simulink model "r_128w_8k_16x_1a_r421"  (Simulink 7.2) (continued)
== system r_128w_8k_16x_1a_r421 == (continued)
      link override convert: arith_type=Unsigned  n_bits=32  bin_pt=0
      mask prompts: io_dir "I/O direction"; arith_type "Data Type"; bitwidth "Data bitwidth"; bin_pt "Data binary point"; sample_period "Sample period"
      mask init:
      | gw_name = clear_name(gcb);
      | config.source = str2func('software_register_init');
      | config.toplevel = gcb;
      | xBlock( config, {io_dir, arith_type, bitwidth, bin_pt, ... 
      |     sample_period, gw_name, latency});
      mask StyleString: popup(From Processor|To Processor),popup(Unsigned|Signed  (2's comp)),edit,edit,edit,edit
  gbe_tx_err_cnt0 : Subsystem  Tag=xps:sw_reg  mask{io_dir=To Processor, arith_type=Unsigned, bitwidth=32, bin_pt=0, sample_period=1, latency=0}
      link override convert: arith_type=Unsigned  n_bits=32  bin_pt=0
      mask prompts: io_dir "I/O direction"; arith_type "Data Type"; bitwidth "Data bitwidth"; bin_pt "Data binary point"; sample_period "Sample period"
      mask init:
      | gw_name = clear_name(gcb);
      | config.source = str2func('software_register_init');
      | config.toplevel = gcb;
      | xBlock( config, {io_dir, arith_type, bitwidth, bin_pt, ... 
      |     sample_period, gw_name, latency});
      mask StyleString: popup(From Processor|To Processor),popup(Unsigned|Signed  (2's comp)),edit,edit,edit,edit
  gpio_tr : Subsystem  mask{cnt_bits=8, time_bits=48}
      mask prompts: cnt_bits "Bits in status counters"; time_bits "No LSBs in time to compare"
  katadc0 : Subsystem  Tag=xps:katadc  AncestorBlock=xps_library/katadc  mask(katadc){adc_brd=adc0, adc_interleave=off, bypass_auto=off, en_gain=on, adc_clk_rate=800, sample_period=1}
      mask prompts: adc_brd "ADC board"; adc_interleave "ADC interleave mode"; bypass_auto "Bypass auto config"; en_gain "Enable gain"; adc_clk_rate "ADC sampling rate (MHz)"; sample_period "Sample period"
      mask init:
      | name = gcb;
      | katadc_init(gcb, ...
      |     'name', name, ...
      |     'adc_brd', adc_brd, ...
      |     'adc_interleave', adc_interleave, ...
      |     'bypass_auto', bypass_auto, ...
      |     'en_gain', en_gain, ...
      |     'adc_clk_rate', adc_clk_rate, ...
      |     'sample_period', sample_period);
      mask StyleString: popup(adc0|adc1),checkbox,checkbox,checkbox,edit,edit
  ld_time_lsw0 : Subsystem  Tag=xps:sw_reg  mask{io_dir=From Processor, arith_type=Unsigned, bitwidth=32, bin_pt=0, sample_period=1, latency=0}
      link override poco_cd_tr0_ld_time_lsb_user_data_out: arith_type=Unsigned  n_bits=32  bin_pt=0  period=1
      link override poco_dram_vacc_r001_cd_tr0_ld_time_lsb_user_data_out: arith_type=Unsigned  n_bits=32  bin_pt=0  period=1
      link override poco_dram_vacc_r002_cd_tr0_ld_time_lsb_user_data_out: arith_type=Unsigned  n_bits=32  bin_pt=0  period=1
      link override poco_dram_vacc_r003_cd_tr0_ld_time_lsb_user_data_out: arith_type=Unsigned  n_bits=32  bin_pt=0  period=1
      link override poco_dram_vacc_r004_cd_tr0_ld_time_lsb_user_data_out: arith_type=Unsigned  n_bits=32  bin_pt=0  period=1
      link override poco_dram_vacc_r005_cd_tr0_ld_time_lsb_user_data_out: arith_type=Unsigned  n_bits=32  bin_pt=0  period=1
      link override poco_dram_vacc_r006_cd_tr0_ld_time_lsb_user_data_out: arith_type=Unsigned  n_bits=32  bin_pt=0  period=1
      link override poco_r006_cd_tr0_ld_time_lsb_user_data_out: arith_type=Unsigned  n_bits=32  bin_pt=0  period=1
      link override poco_red_cd_ld_time_lsb_user_data_out: arith_type=Unsigned  n_bits=32  bin_pt=0  period=1
      link override poco_ref_roach_adc0_cd_ld_time_lsb_user_data_out: arith_type=Unsigned  n_bits=32  bin_pt=0  period=1
      link override poco_ref_roach_reduced_adc0_cd_ld_time_lsb_user_data_out: arith_type=Unsigned  n_bits=32  bin_pt=0  period=1
      link override poco_roach_cd_tr0_ld_time_lsb_user_data_out: arith_type=Unsigned  n_bits=32  bin_pt=0  period=1
      link override poco_roach_r006_cd_tr0_ld_time_lsb_user_data_out: arith_type=Unsigned  n_bits=32  bin_pt=0  period=1
      link override poco_roach_r007_cd_tr0_ld_time_lsb_user_data_out: arith_type=Unsigned  n_bits=32  bin_pt=0  period=1
      link override poco_roach_red_adc0_cd_ld_time_lsb_user_data_out: arith_type=Unsigned  n_bits=32  bin_pt=0  period=1
      link override poco_roach_vacc_r006_cd_tr0_ld_time_lsb_user_data_out: arith_type=Unsigned  n_bits=32  bin_pt=0  period=1
      mask prompts: io_dir "I/O direction"; arith_type "Data Type"; bitwidth "Data bitwidth"; bin_pt "Data binary point"; sample_period "Sample period"
      mask init:
      | gw_name = clear_name(gcb);
      | config.source = str2func('software_register_init');
      | config.toplevel = gcb;
      | xBlock( config, {io_dir, arith_type, bitwidth, bin_pt, ... 
      |     sample_period, gw_name, latency});
      mask StyleString: popup(From Processor|To Processor),popup(Unsigned|Signed  (2's comp)),edit,edit,edit,edit
  ld_time_lsw1 : Subsystem  Tag=xps:sw_reg  mask{io_dir=From Processor, arith_type=Unsigned, bitwidth=32, bin_pt=0, sample_period=1, latency=0}
      link override poco_cd_tr0_ld_time_lsb_user_data_out: arith_type=Unsigned  n_bits=32  bin_pt=0  period=1
      link override poco_dram_vacc_r001_cd_tr0_ld_time_lsb_user_data_out: arith_type=Unsigned  n_bits=32  bin_pt=0  period=1
      link override poco_dram_vacc_r002_cd_tr0_ld_time_lsb_user_data_out: arith_type=Unsigned  n_bits=32  bin_pt=0  period=1
      link override poco_dram_vacc_r003_cd_tr0_ld_time_lsb_user_data_out: arith_type=Unsigned  n_bits=32  bin_pt=0  period=1
      link override poco_dram_vacc_r004_cd_tr0_ld_time_lsb_user_data_out: arith_type=Unsigned  n_bits=32  bin_pt=0  period=1
      link override poco_dram_vacc_r005_cd_tr0_ld_time_lsb_user_data_out: arith_type=Unsigned  n_bits=32  bin_pt=0  period=1
      link override poco_dram_vacc_r006_cd_tr0_ld_time_lsb_user_data_out: arith_type=Unsigned  n_bits=32  bin_pt=0  period=1
      link override poco_r006_cd_tr0_ld_time_lsb_user_data_out: arith_type=Unsigned  n_bits=32  bin_pt=0  period=1
      link override poco_red_cd_ld_time_lsb_user_data_out: arith_type=Unsigned  n_bits=32  bin_pt=0  period=1
      link override poco_ref_roach_adc0_cd_ld_time_lsb_user_data_out: arith_type=Unsigned  n_bits=32  bin_pt=0  period=1
      link override poco_ref_roach_reduced_adc0_cd_ld_time_lsb_user_data_out: arith_type=Unsigned  n_bits=32  bin_pt=0  period=1
      link override poco_roach_cd_tr0_ld_time_lsb_user_data_out: arith_type=Unsigned  n_bits=32  bin_pt=0  period=1
      link override poco_roach_r006_cd_tr0_ld_time_lsb_user_data_out: arith_type=Unsigned  n_bits=32  bin_pt=0  period=1
      link override poco_roach_r007_cd_tr0_ld_time_lsb_user_data_out: arith_type=Unsigned  n_bits=32  bin_pt=0  period=1
      link override poco_roach_red_adc0_cd_ld_time_lsb_user_data_out: arith_type=Unsigned  n_bits=32  bin_pt=0  period=1
      link override poco_roach_vacc_r006_cd_tr0_ld_time_lsb_user_data_out: arith_type=Unsigned  n_bits=32  bin_pt=0  period=1
      mask prompts: io_dir "I/O direction"; arith_type "Data Type"; bitwidth "Data bitwidth"; bin_pt "Data binary point"; sample_period "Sample period"
      mask init:
      | gw_name = clear_name(gcb);
      | config.source = str2func('software_register_init');
      | config.toplevel = gcb;
      | xBlock( config, {io_dir, arith_type, bitwidth, bin_pt, ... 
      |     sample_period, gw_name, latency});
      mask StyleString: popup(From Processor|To Processor),popup(Unsigned|Signed  (2's comp)),edit,edit,edit,edit
  ld_time_msw0 : Subsystem  Tag=xps:sw_reg  mask{io_dir=From Processor, arith_type=Unsigned, bitwidth=32, bin_pt=0, sample_period=1, latency=0}
      link override poco_cd_tr0_ld_time_msb_user_data_out: arith_type=Unsigned  n_bits=32  bin_pt=0  period=1
      link override poco_dram_vacc_r001_cd_tr0_ld_time_msb_user_data_out: arith_type=Unsigned  n_bits=32  bin_pt=0  period=1
      link override poco_dram_vacc_r002_cd_tr0_ld_time_msb_user_data_out: arith_type=Unsigned  n_bits=32  bin_pt=0  period=1
      link override poco_dram_vacc_r003_cd_tr0_ld_time_msb_user_data_out: arith_type=Unsigned  n_bits=32  bin_pt=0  period=1
      link override poco_dram_vacc_r004_cd_tr0_ld_time_msb_user_data_out: arith_type=Unsigned  n_bits=32  bin_pt=0  period=1
      link override poco_dram_vacc_r005_cd_tr0_ld_time_msb_user_data_out: arith_type=Unsigned  n_bits=32  bin_pt=0  period=1
      link override poco_dram_vacc_r006_cd_tr0_ld_time_msb_user_data_out: arith_type=Unsigned  n_bits=32  bin_pt=0  period=1
      link override poco_r006_cd_tr0_ld_time_msb_user_data_out: arith_type=Unsigned  n_bits=32  bin_pt=0  period=1
      link override poco_red_cd_ld_time_msb_user_data_out: arith_type=Unsigned  n_bits=32  bin_pt=0  period=1
      link override poco_ref_roach_adc0_cd_ld_time_msb_user_data_out: arith_type=Unsigned  n_bits=32  bin_pt=0  period=1
      link override poco_ref_roach_reduced_adc0_cd_ld_time_msb_user_data_out: arith_type=Unsigned  n_bits=32  bin_pt=0  period=1
      link override poco_roach_cd_tr0_ld_time_msb_user_data_out: arith_type=Unsigned  n_bits=32  bin_pt=0  period=1
      link override poco_roach_r006_cd_tr0_ld_time_msb_user_data_out: arith_type=Unsigned  n_bits=32  bin_pt=0  period=1
      link override poco_roach_r007_cd_tr0_ld_time_msb_user_data_out: arith_type=Unsigned  n_bits=32  bin_pt=0  period=1
      link override poco_roach_red_adc0_cd_ld_time_msb_user_data_out: arith_type=Unsigned  n_bits=32  bin_pt=0  period=1
      link override poco_roach_vacc_r006_cd_tr0_ld_time_msb_user_data_out: arith_type=Unsigned  n_bits=32  bin_pt=0  period=1
      mask prompts: io_dir "I/O direction"; arith_type "Data Type"; bitwidth "Data bitwidth"; bin_pt "Data binary point"; sample_period "Sample period"
      mask init:
      | gw_name = clear_name(gcb);
      | config.source = str2func('software_register_init');
      | config.toplevel = gcb;
      | xBlock( config, {io_dir, arith_type, bitwidth, bin_pt, ... 
      |     sample_period, gw_name, latency});
      mask StyleString: popup(From Processor|To Processor),popup(Unsigned|Signed  (2's comp)),edit,edit,edit,edit
  ld_time_msw1 : Subsystem  Tag=xps:sw_reg  mask{io_dir=From Processor, arith_type=Unsigned, bitwidth=32, bin_pt=0, sample_period=1, latency=0}
      link override poco_cd_tr0_ld_time_msb_user_data_out: arith_type=Unsigned  n_bits=32  bin_pt=0  period=1
      link override poco_dram_vacc_r001_cd_tr0_ld_time_msb_user_data_out: arith_type=Unsigned  n_bits=32  bin_pt=0  period=1
      link override poco_dram_vacc_r002_cd_tr0_ld_time_msb_user_data_out: arith_type=Unsigned  n_bits=32  bin_pt=0  period=1
      link override poco_dram_vacc_r003_cd_tr0_ld_time_msb_user_data_out: arith_type=Unsigned  n_bits=32  bin_pt=0  period=1
      link override poco_dram_vacc_r004_cd_tr0_ld_time_msb_user_data_out: arith_type=Unsigned  n_bits=32  bin_pt=0  period=1
      link override poco_dram_vacc_r005_cd_tr0_ld_time_msb_user_data_out: arith_type=Unsigned  n_bits=32  bin_pt=0  period=1
      link override poco_dram_vacc_r006_cd_tr0_ld_time_msb_user_data_out: arith_type=Unsigned  n_bits=32  bin_pt=0  period=1
      link override poco_r006_cd_tr0_ld_time_msb_user_data_out: arith_type=Unsigned  n_bits=32  bin_pt=0  period=1
      link override poco_red_cd_ld_time_msb_user_data_out: arith_type=Unsigned  n_bits=32  bin_pt=0  period=1
      link override poco_ref_roach_adc0_cd_ld_time_msb_user_data_out: arith_type=Unsigned  n_bits=32  bin_pt=0  period=1
      link override poco_ref_roach_reduced_adc0_cd_ld_time_msb_user_data_out: arith_type=Unsigned  n_bits=32  bin_pt=0  period=1
      link override poco_roach_cd_tr0_ld_time_msb_user_data_out: arith_type=Unsigned  n_bits=32  bin_pt=0  period=1
      link override poco_roach_r006_cd_tr0_ld_time_msb_user_data_out: arith_type=Unsigned  n_bits=32  bin_pt=0  period=1
      link override poco_roach_r007_cd_tr0_ld_time_msb_user_data_out: arith_type=Unsigned  n_bits=32  bin_pt=0  period=1
      link override poco_roach_red_adc0_cd_ld_time_msb_user_data_out: arith_type=Unsigned  n_bits=32  bin_pt=0  period=1
      link override poco_roach_vacc_r006_cd_tr0_ld_time_msb_user_data_out: arith_type=Unsigned  n_bits=32  bin_pt=0  period=1
      mask prompts: io_dir "I/O direction"; arith_type "Data Type"; bitwidth "Data bitwidth"; bin_pt "Data binary point"; sample_period "Sample period"
      mask init:
      | gw_name = clear_name(gcb);
      | config.source = str2func('software_register_init');
      | config.toplevel = gcb;
      | xBlock( config, {io_dir, arith_type, bitwidth, bin_pt, ... 
      |     sample_period, gw_name, latency});
      mask StyleString: popup(From Processor|To Processor),popup(Unsigned|Signed  (2's comp)),edit,edit,edit,edit
  leds : Subsystem
  man_sync1 : xbsIndex_r4/Slice  nbits=1  boolean_output=on  mode=Lower Bit Location + Width  bit1=0  base1=MSB of Input  bit0=1  base0=LSB of Input  dbl_ovrd=off  block_type=slice
      settings: block_version=10.1.3
  mcount_lsw : Subsystem  Tag=xps:sw_reg  mask{io_dir=To Processor, arith_type=Unsigned, bitwidth=32, bin_pt=0, sample_period=1, latency=0}
      link override convert: arith_type=Unsigned  n_bits=32  bin_pt=0
      mask prompts: io_dir "I/O direction"; arith_type "Data Type"; bitwidth "Data bitwidth"; bin_pt "Data binary point"; sample_period "Sample period"
      mask init:
      | gw_name = clear_name(gcb);
      | config.source = str2func('software_register_init');
      | config.toplevel = gcb;
      | xBlock( config, {io_dir, arith_type, bitwidth, bin_pt, ... 
      |     sample_period, gw_name, latency});
      mask StyleString: popup(From Processor|To Processor),popup(Unsigned|Signed  (2's comp)),edit,edit,edit,edit
  mcount_msw : Subsystem  Tag=xps:sw_reg  mask{io_dir=To Processor, arith_type=Unsigned, bitwidth=32, bin_pt=0, sample_period=1, latency=0}
      link override convert: arith_type=Unsigned  n_bits=32  bin_pt=0
      mask prompts: io_dir "I/O direction"; arith_type "Data Type"; bitwidth "Data bitwidth"; bin_pt "Data binary point"; sample_period "Sample period"
      mask init:
      | gw_name = clear_name(gcb);
      | config.source = str2func('software_register_init');
      | config.toplevel = gcb;
      | xBlock( config, {io_dir, arith_type, bitwidth, bin_pt, ... 
      |     sample_period, gw_name, latency});
      mask StyleString: popup(From Processor|To Processor),popup(Unsigned|Signed  (2's comp)),edit,edit,edit,edit
  packetiser : Subsystem  mask{payload_bits=5, xengine_bits=4, fft_bits=13}
      mask prompts: payload_bits "Payload length in 64bit words (2^?)"; xengine_bits "Number of x-engines (2^?)"; fft_bits "Number of frequencies (2^?)"
      mask help: Package 32 bit data into 64 bits and identify data as coming from antenna 0 or 1
  pfb_fir_real0 : Subsystem  AncestorBlock=casper_library_pfbs/pfb_fir_real  mask(pfb_fir_real){PFBSize=14, TotalTaps=4, WindowType=hamming, n_inputs=2, MakeBiplex=0, BitWidthIn=8, BitWidthOut=18, CoeffBitWidth=18, CoeffDistMem=0, add_latency=1, mult_latency=2, bram_latency=2, conv_latency=2, quantization=Round  (unbiased: +/- Inf), fwidth=1, specify_mult=off, mult_spec=[2                                      2], adder_folding=on, adder_imp=Behavioral}
      mask prompts: PFBSize "Size of PFB: (2^? pnts)"; TotalTaps "Total Number of Taps:"; WindowType "Windowing Function:"; n_inputs "Number of Simultaneous Inputs: (2^?)"; MakeBiplex "Make Biplex"; BitWidthIn "Input Bitwidth:"; BitWidthOut "Output Bitwidth:"; CoeffBitWidth "Coefficient Bitwidth:"; CoeffDistMem "Use Distributed Memory for Coeffs"; add_latency "Add Latency"; mult_latency "Mult Latency"; bram_latency "BRAM Latency"; conv_latency "Convert latency"; quantization "Quantization Behavior"; fwidth "Bin Width Scaling (normal=1)"; specify_mult "Specify multiplier use (behavioral if not specified)"; mult_spec "Multiplier specification (0=core, 1=embedded, 2=behavioural) (left=1st tap)"; adder_folding "Fold adders into DSPs"; adder_imp "Adder implementation"
      mask help:
      | Fold adders into DSPs: Causes adders to be absorbed into DSP blocks (supported in Virtex5)
      | Adder implementation: Cores using Fabric or DSP48 or behavioral HDL
      mask init:
      | pfb_fir_real_init(gcb,...
      |     'PFBSize', PFBSize,...
      |     'TotalTaps', TotalTaps,...
      |     'WindowType', WindowType,...
      |     'n_inputs', n_inputs,...
      |     'MakeBiplex', MakeBiplex,...
      |     'BitWidthIn', BitWidthIn,...
      |     'BitWidthOut', BitWidthOut,...
      |     'CoeffBitWidth', CoeffBitWidth,...
      |     'CoeffDistMem', CoeffDistMem,...
      |     'add_latency', add_latency,...
      |     'mult_latency', mult_latency,...
      |     'bram_latency', bram_latency,...
      |     'conv_latency', conv_latency,...
      |     'quantization', quantization,...
      |     'fwidth', fwidth,...
      |     'specify_mult', specify_mult,...
      |     'mult_spec', mult_spec,...
      |     'adder_folding', adder_folding, ...
      |     'adder_imp', adder_imp);
      settings: AttributesFormatString=taps=4, add_latency=1
      mask Help: eval('xlWeb(''http://casper.berkeley.edu/wiki/Pfb_fir_real'')')
      mask StyleString: edit,edit,popup(bartlett|barthannwin|blackman|blackmanharris|bohamwin|chebwin|flattopwin|gausswin|hamming|hann|kaiser|nuttallwin|parzenwin|rectwin|tukeywin|triang|userwindow),edit,edit,edit,edit,edit,edit,edit,edit,edit,edit,popup(Truncate|Round  (unbiased: +/- Inf)|Round  (unbiased: Even Values)),edit,checkbox,edit,checkbox,popup(Behavioral|Fabric|DSP48)
      mask CallbackString:
      | |%num_taps = eval(get_param(gcb, 'TotalTaps'));
      | %old_vec = eval(get_param(gcb, 'mult_spec'));=-0l
      | 
      | %vec = 2.*ones(1,num_taps);
      | %old_size = length(old_vec);
      | 
      | %if(old_size <= num_taps),
      | %    new_size = old_size;
      | %else,
      | %    new_size = num_taps;
      | %end
      | 
      | %for n = 1:new_size, vec(n) = old_vec(n); end
      | %set_param(gcb, 'mult_spec', mat2str(vec));||||||||||||||%thisVariable = 'specify_mult';
      | %affectedVars = {'mult_spec'};
      | 
      | %thisBlock = gcb();
      | %thisVarState = get_param(thisBlock, thisVariable);
      | %maskNames = get_param(thisBlock, 'MaskNames');
      | %maskEnables = get_param(thisBlock, 'MaskEnables');
      | 
      | %for varName = affectedVars
      | %    varIndex = find(ismember(maskNames, varName));
      | %    maskEnables{varIndex} = thisVarState;
      | %end
      | 
      | %set_param(thisBlock, 'MaskEnables', maskEnables);|||
  pfb_fir_real1 : Subsystem  AncestorBlock=casper_library_pfbs/pfb_fir_real  mask(pfb_fir_real){PFBSize=14, TotalTaps=4, WindowType=hamming, n_inputs=2, MakeBiplex=0, BitWidthIn=8, BitWidthOut=18, CoeffBitWidth=18, CoeffDistMem=0, add_latency=1, mult_latency=2, bram_latency=2, conv_latency=2, quantization=Round  (unbiased: +/- Inf), fwidth=1, specify_mult=off, mult_spec=[2                                      2], adder_folding=on, adder_imp=Behavioral}
      mask prompts: PFBSize "Size of PFB: (2^? pnts)"; TotalTaps "Total Number of Taps:"; WindowType "Windowing Function:"; n_inputs "Number of Simultaneous Inputs: (2^?)"; MakeBiplex "Make Biplex"; BitWidthIn "Input Bitwidth:"; BitWidthOut "Output Bitwidth:"; CoeffBitWidth "Coefficient Bitwidth:"; CoeffDistMem "Use Distributed Memory for Coeffs"; add_latency "Add Latency"; mult_latency "Mult Latency"; bram_latency "BRAM Latency"; conv_latency "Convert latency"; quantization "Quantization Behavior"; fwidth "Bin Width Scaling (normal=1)"; specify_mult "Specify multiplier use (behavioral if not specified)"; mult_spec "Multiplier specification (0=core, 1=embedded, 2=behavioural) (left=1st tap)"; adder_folding "Fold adders into DSPs"; adder_imp "Adder implementation"
      mask help:
      | Fold adders into DSPs: Causes adders to be absorbed into DSP blocks (supported in Virtex5)
      | Adder implementation: Cores using Fabric or DSP48 or behavioral HDL
      mask init: same as r_128w_8k_16x_1a_r421/pfb_fir_real0
      settings: AttributesFormatString=taps=4, add_latency=1
      mask Help: eval('xlWeb(''http://casper.berkeley.edu/wiki/Pfb_fir_real'')')
      mask StyleString: edit,edit,popup(bartlett|barthannwin|blackman|blackmanharris|bohamwin|chebwin|flattopwin|gausswin|hamming|hann|kaiser|nuttallwin|parzenwin|rectwin|tukeywin|triang|userwindow),edit,edit,edit,edit,edit,edit,edit,edit,edit,edit,popup(Truncate|Round  (unbiased: +/- Inf)|Round  (unbiased: Even Values)),edit,checkbox,edit,checkbox,popup(Behavioral|Fabric|DSP48)
      mask CallbackString:
      | |%num_taps = eval(get_param(gcb, 'TotalTaps'));
      | %old_vec = eval(get_param(gcb, 'mult_spec'));=-0l
      | 
      | %vec = 2.*ones(1,num_taps);
      | %old_size = length(old_vec);
      | 
      | %if(old_size <= num_taps),
      | %    new_size = old_size;
      | %else,
      | %    new_size = num_taps;
      | %end
      | 
      | %for n = 1:new_size, vec(n) = old_vec(n); end
      | %set_param(gcb, 'mult_spec', mat2str(vec));||||||||||||||%thisVariable = 'specify_mult';
      | %affectedVars = {'mult_spec'};
      | 
      | %thisBlock = gcb();
      | %thisVarState = get_param(thisBlock, thisVariable);
      | %maskNames = get_param(thisBlock, 'MaskNames');
      | %maskEnables = get_param(thisBlock, 'MaskEnables');
      | 
      | %for varName = affectedVars
      | %    varIndex = find(ismember(maskNames, varName));
      | %    maskEnables{varIndex} = thisVarState;
      | %end
      | 
      | %set_param(thisBlock, 'MaskEnables', maskEnables);|||
  pkt_cont_tvg0 : Subsystem  mask{p_bits=5, fft_bits=13, x_bits=4}
      mask prompts: p_bits "packet size (2^? 64 bit words)"; fft_bits "Number of channels (2^?)"; x_bits "n_x_engines (2^?)"
  pol_pack0 : Subsystem
  pol_pack1 : Subsystem
  posedge{1..2,23..24} (4 alike) : casper_library_misc/posedge  Tag=Rising Edge Detector  SystemSampleTime=-1
  pps_count : Subsystem  Tag=xps:sw_reg  mask{io_dir=To Processor, arith_type=Unsigned, bitwidth=32, bin_pt=0, sample_period=1, latency=0}
      link override convert: arith_type=Unsigned  n_bits=32  bin_pt=0
      mask prompts: io_dir "I/O direction"; arith_type "Data Type"; bitwidth "Data bitwidth"; bin_pt "Data binary point"; sample_period "Sample period"
      mask init:
      | gw_name = clear_name(gcb);
      | config.source = str2func('software_register_init');
      | config.toplevel = gcb;
      | xBlock( config, {io_dir, arith_type, bitwidth, bin_pt, ... 
      |     sample_period, gw_name, latency});
      mask StyleString: popup(From Processor|To Processor),popup(Unsigned|Signed  (2's comp)),edit,edit,edit,edit
  quant0 : Subsystem  mask{channel_bits=13, din_bit_width=18, din_bin_pt=17, coeff_bit_width=16, coeff_bin_pt=1, dout_bit_width=4, dout_bin_pt=3, init_val=4, overflow=Saturate, quantization=Round  (unbiased: even values), conv_latency=2, add_latency=1, mult_latency=2, bram_latency=2}
      mask prompts: channel_bits "Number channels (2^?)"; din_bit_width "Data input bit width"; din_bin_pt "Data input binary point"; coeff_bit_width "Coefficient bit width"; coeff_bin_pt "Coefficient binary point"; dout_bit_width "Data out bit width"; dout_bin_pt "Data out binary point"; init_val "Initial value"; overflow "Overflow behaviour"; quantization "Quantization Behaviour"; conv_latency "Conversion latency"; add_latency "Adder latency"; mult_latency "Multiplier latency"; bram_latency "BRAM latency"
      mask init:
      | blk = gcb;
      | set_param([blk,'/convert_of0'], ...
      |     'bit_width_i', num2str(din_bit_width+coeff_bit_width+1), ...
      |     'binary_point_i', num2str(din_bin_pt+coeff_bin_pt), ...
      |     'bit_width_o', num2str(dout_bit_width), 'binary_point_o', num2str(dout_bin_pt), ...
      |     'latency', num2str(conv_latency), 'overflow', overflow, ...
      |     'quantization', quantization);
      | set_param([blk,'/convert_of1'], ...
      |     'bit_width_i', num2str(din_bit_width+coeff_bit_width+1), ...
      |     'binary_point_i', num2str(din_bin_pt+coeff_bin_pt), ...
      |     'bit_width_o', num2str(dout_bit_width), 'binary_point_o', num2str(dout_bin_pt), ...
      |     'latency', num2str(conv_latency), 'overflow', overflow, ...
      |     'quantization', quantization);
      | set_param([blk,'/convert_of2'], ...
      |     'bit_width_i', num2str(din_bit_width+coeff_bit_width+1), ...
      |     'binary_point_i', num2str(din_bin_pt+coeff_bin_pt), ...
      |     'bit_width_o', num2str(dout_bit_width), 'binary_point_o', num2str(dout_bin_pt), ...
      |     'latency', num2str(conv_latency), 'overflow', overflow, ...
      |     'quantization', quantization);
      | set_param([blk,'/convert_of3'], ...
      |     'bit_width_i', num2str(din_bit_width+coeff_bit_width+1), ...
      |     'binary_point_i', num2str(din_bin_pt+coeff_bin_pt), ...
      |     'bit_width_o', num2str(dout_bit_width), 'binary_point_o', num2str(dout_bin_pt), ...
      |     'latency', num2str(conv_latency), 'overflow', overflow, ...
      |     'quantization', quantization);
      mask StyleString: edit,edit,edit,edit,edit,edit,edit,edit,popup(Wrap|Saturate|Error),popup(Truncate|Round  (unbiased: +/- Inf)|Round  (unbiased: even values)),edit,edit,edit,edit
  quant1 : Subsystem  mask{channel_bits=13, din_bit_width=18, din_bin_pt=17, coeff_bit_width=16, coeff_bin_pt=1, dout_bit_width=4, dout_bin_pt=3, init_val=4, overflow=Saturate, quantization=Round  (unbiased: even values), conv_latency=2, add_latency=1, mult_latency=2, bram_latency=2}
      mask prompts: channel_bits "Number channels (2^?)"; din_bit_width "Data input bit width"; din_bin_pt "Data input binary point"; coeff_bit_width "Coefficient bit width"; coeff_bin_pt "Coefficient binary point"; dout_bit_width "Data out bit width"; dout_bin_pt "Data out binary point"; init_val "Initial value"; overflow "Overflow behaviour"; quantization "Quantization Behaviour"; conv_latency "Conversion latency"; add_latency "Adder latency"; mult_latency "Multiplier latency"; bram_latency "BRAM latency"
      mask init: same as r_128w_8k_16x_1a_r421/quant0
      mask StyleString: edit,edit,edit,edit,edit,edit,edit,edit,popup(Wrap|Saturate|Error),popup(Truncate|Round  (unbiased: +/- Inf)|Round  (unbiased: even values)),edit,edit,edit,edit
  rcs : Subsystem  AncestorBlock=casper_library_misc/rcs  mask(rcs){app_src=git, lib_src=git, user_val=421}
      mask prompts: app_src "Application revision info to capture"; lib_src "CASPER libraries revision info to capture"; user_val "User specified value"
      mask help:
      | Embeds revision control information for the user design and libraries.
      | On failure to locate specified revision information, falls back to
      | timestamps (current time for libraries).
      | A user specified value is also stored.
      mask init:
      | rcs_init(gcb, ...
      |     'app_src', app_src, ...
      |     'lib_src', lib_src);
      mask StyleString: popup(timestamp|git|svn),popup(timestamp|git|svn),edit
  scopes : Subsystem
  snap_gbe_tx0 : Subsystem  AncestorBlock=casper_library_scopes/snapshot  mask(snapshot){storage=bram, dram_dimm=1, dram_clock=200, nsamples=8, data_width=128, offset=on, circap=off, value=off, use_dsp48=off}
      mask prompts: storage "Storage medium"; dram_dimm "DRAM dimm"; dram_clock "DRAM clock rate"; nsamples "Number of Samples (2^?)"; data_width "Data width"; offset "Start delay support"; circap "Circular capture support"; value "Extra value capture support"; use_dsp48 "Use DSP48s to implement counters"
      mask help:
      | Standard snap block, but with the following optional extras;
      |  1) Optionally delay the fabric trigger by a user-specified number of valids.
      |  2) Capture continuously after a trigger until a stop pulse is received. Data is captured into BRAM continuously, with old data being overwritten until a stop command is received. Then stop capturing when memory is filled. This enables you to get some data before and some data after the stop pulse. Use the 'tr_en_cnt' register to determine where you are in your vector. 'Addr' register's MSb now indicates 'busy capturing'.
      |  3) Optionally capture a value on an input port to a register at the same time as the first data sample.
      |  4) Implement counters using DSP48Es to save resources.
      mask init: same as r_128w_8k_16x_1a_r421/adc_snap0
      mask StyleString: popup(bram|dram),popup(1|2|3|4),popup(200|250|266|300|333),edit,popup(8|16|32|64|128),checkbox,checkbox,checkbox,checkbox
      mask CallbackString: snapshot_callback();||||||||
  timing : Subsystem  mask{reset_period=1, sync_gen_bits=28}
      mask prompts: reset_period "Master reset time (sync pulses)"; sync_gen_bits "Size of sync generator counter (bits)"
  trig0 : Subsystem  mask{mult_latency=3}
      mask prompts: mult_latency "Multiplier latency"
  trig1 : Subsystem  mask{mult_latency=3}
      mask prompts: mult_latency "Multiplier latency"
  trig_level : Subsystem  Tag=xps:sw_reg  mask{io_dir=From Processor, arith_type=Unsigned, bitwidth=32, bin_pt=0, sample_period=1, latency=0}
      link override convert: arith_type=Unsigned  n_bits=32  bin_pt=0
      link override r_spec_2048_r102_cnt_rst_user_data_out: arith_type=Unsigned  n_bits=32  bin_pt=0  period=1
      link override r_spec_2048_r102_nosim_cnt_rst_user_data_out: arith_type=Unsigned  n_bits=32  bin_pt=0  period=1
      link override r_spec_2048_r103_cnt_rst_user_data_out: arith_type=Unsigned  n_bits=32  bin_pt=0  period=1
      link override r_spec_2048_r104_cnt_rst_user_data_out: arith_type=Unsigned  n_bits=32  bin_pt=0  period=1
      link override r_spec_2048_r104_test0_cnt_rst_user_data_out: arith_type=Unsigned  n_bits=32  bin_pt=0  period=1
      link override r_spec_2048_r104_test_cnt_rst_user_data_out: arith_type=Unsigned  n_bits=32  bin_pt=0  period=1
      link override r_spec_test0_cnt_rst_user_data_out: arith_type=Unsigned  n_bits=32  bin_pt=0  period=1
      link override r_spec_test4_cnt_rst_user_data_out: arith_type=Unsigned  n_bits=32  bin_pt=0  period=1
      link override roach_spec_no10gbe_sync_cnt_rst_user_data_out: arith_type=Unsigned  n_bits=32  bin_pt=0  period=1
      link override roach_spec_rst_cnt_user_data_out: arith_type=Unsigned  n_bits=32  bin_pt=0  period=1
      link override roach_spec_rst_sync_cnt_user_data_out: arith_type=Unsigned  n_bits=32  bin_pt=0  period=1
      mask prompts: io_dir "I/O direction"; arith_type "Data Type"; bitwidth "Data bitwidth"; bin_pt "Data binary point"; sample_period "Sample period"
      mask init:
      | gw_name = clear_name(gcb);
      | config.source = str2func('software_register_init');
      | config.toplevel = gcb;
      | xBlock( config, {io_dir, arith_type, bitwidth, bin_pt, ... 
      |     sample_period, gw_name, latency});
      mask StyleString: popup(From Processor|To Processor),popup(Unsigned|Signed  (2's comp)),edit,edit,edit,edit
  tvg_tr : Subsystem  mask{cnt_bits=8, time_bits=48}
      mask prompts: cnt_bits "Bits in status counters"; time_bits "No LSBs in time to compare"
  xaui_pack0 : Subsystem  mask{payload_len=32, xeng_bits=4, chan_bits=13, skip_xeng_bits=1}
      mask prompts: payload_len "Payload Length (in 64 bit words)"; xeng_bits "Number of X engines (2^?)"; chan_bits "Number of Frequency channels (2^?)"; skip_xeng_bits "X engines per IP address (2^?)"
      mask help: Packetizes data coming in over a XAUI interface. A packet consists of a 64 bit header (48 bits of "mcnt" and 16 bits of antenna), followed by 64 x "payload" bits of payload. "Mcnt" (master count) is a counter which keeps track of how many packets have been transmitted since the last "mrst".
      mask init:
      | packet_len = payload_len + 1;
      | packet_bits = ceil(log2(packet_len));
      | payload_bits = ceil(log2(payload_len));
  wires:
  [adc_sync0] -> Logical3/2
  timing/2(sync) -> [sync]
  Logical3/1 -> timing/4(pps_in), [pps]
  Concat/1 -> timing/2(data_in)
  Constant9/1 -> fft_shift0/1(sim_reg_in)
  fft_shift0/1(reg_in) -> Slice6/1
  a0p0_1st_shift/2(dout1) -> fft_wideband_real0/3(in0)
  a0p0_1st_shift/3(dout2) -> fft_wideband_real0/4(in1)
  a0p0_1st_shift/4(dout3) -> fft_wideband_real0/5(in2)
  a0p0_1st_shift/5(dout4) -> fft_wideband_real0/6(in3)
  Slice6/1 -> fft_wideband_real0/2(shift)
  a0p0_1st_shift/1(sync_out) -> fft_wideband_real0/1(sync)
  fft_wideband_real0/1(sync_out) -> fd_local_time/2(sync), Delay20/1
  fft_wideband_real0/4(of) -> [fft_or0]
  pfb_fir_real1/2(pol1_out1) -> a0p1_1st_shift/2(din1)
  pfb_fir_real1/3(pol1_out2) -> a0p1_1st_shift/3(din2)
  pfb_fir_real1/4(pol1_out3) -> a0p1_1st_shift/4(din3)
  pfb_fir_real1/5(pol1_out4) -> a0p1_1st_shift/5(din4)
  a0p1_1st_shift/2(dout1) -> fft_wideband_real1/3(in0)
  a0p1_1st_shift/3(dout2) -> fft_wideband_real1/4(in1)
  a0p1_1st_shift/4(dout3) -> fft_wideband_real1/5(in2)
  a0p1_1st_shift/5(dout4) -> fft_wideband_real1/6(in3)
  pfb_fir_real1/1(sync_out) -> a0p1_1st_shift/1(sync_in)
  a0p1_1st_shift/1(sync_out) -> fft_wideband_real1/1(sync)
  [sync] -> pfb_fir_real1/1(sync)
  bus_expand5/1(msb_out4) -> pfb_fir_real1/2(pol1_in1)
  bus_expand5/2(out3) -> pfb_fir_real1/3(pol1_in2)
  bus_expand5/3(out2) -> pfb_fir_real1/4(pol1_in3)
  bus_expand5/4(lsb_out1) -> pfb_fir_real1/5(pol1_in4)
  bus_expand7/1(msb_out4) -> pfb_fir_real0/2(pol1_in1)
  bus_expand7/2(out3) -> pfb_fir_real0/3(pol1_in2)
  bus_expand7/3(out2) -> pfb_fir_real0/4(pol1_in3)
  bus_expand7/4(lsb_out1) -> pfb_fir_real0/5(pol1_in4)
  quant0/2(dout0) -> Concat7/1
  quant0/3(dout1) -> Concat7/2
  quant0/1(sync_out) -> [quant0_sync]
  quant0/4(of) -> [quant_or0]
  Concat7/1 -> [quant0_data]
  timing/1(armed) -> Logical14/2, [armed]
  Logical14/1 -> [mrst]
  [mrst] -> cd_local_time/1(reset)
  [sync] -> cd_local_time/2(sync)
  cd_local_time/1(time) -> [cd_local_time]
  [sys_rst] -> Logical14/1
  [man_sync] -> Logical3/1
  [arm] -> timing/1(arm)
  Constant2/1 -> timing/3(sync_period)
  pfb_fir_real0/2(pol1_out1) -> a0p0_1st_shift/2(din1)
  pfb_fir_real0/3(pol1_out2) -> a0p0_1st_shift/3(din2)
  pfb_fir_real0/4(pol1_out3) -> a0p0_1st_shift/4(din3)
  pfb_fir_real0/5(pol1_out4) -> a0p0_1st_shift/5(din4)
  pfb_fir_real0/1(sync_out) -> a0p0_1st_shift/1(sync_in)
  Constant33/1 -> ld_time_msw0/1(sim_reg_in)
  Constant29/1 -> ld_time_lsw0/1(sim_reg_in)
  ld_time_msw0/1(reg_in) -> Slice73/1, Slice7/1
  Slice73/1 -> Concat9/1
  ld_time_lsw0/1(reg_in) -> Concat9/2
  fstatus0/1(sim_reg_out) -> Terminator9/1
  Constant18/1 -> control/1(sim_reg_in)
  control/1(reg_in) -> Delay6/1
  Delay6/1 -> Slice78/1, man_sync1/1, arm1/1, arm2/1, arm8/1, arm10/1, arm6/1, arm7/1, arm5/1, arm4/1, arm3/1, arm11/1, arm9/1
  Slice78/1 -> [sys_rst]
  man_sync1/1 -> posedge1/1
  posedge1/1 -> [man_sync]
  arm1/1 -> posedge24/1
  posedge24/1 -> [arm]
  arm2/1 -> posedge23/1
  posedge23/1 -> [clr_status]
  Counter1/1 -> Register19/1
  Register19/1 -> clk_frequency/1(reg_out), Relational/1, Relational1/1
  clk_frequency/1(sim_reg_out) -> Terminator10/1
  pps_count/1(sim_reg_out) -> Terminator11/1
  [mrst] -> Counter3/1
  [cd_local_time] -> Delay7/1
  mcount_msw/1(sim_reg_out) -> Terminator12/1
  mcount_lsw/1(sim_reg_out) -> Terminator13/1
  Slice/1 -> mcount_lsw/1(reg_out)
  Slice1/1 -> mcount_msw/1(reg_out)
  fstatus1/1(sim_reg_out) -> Terminator2/1
  Constant32/1 -> coarse_delay0/1(sim_reg_in)
  Constant8/1 -> a0_fd0/1(sim_reg_in)
  Constant4/1 -> a1_fd0/1(sim_reg_in)
  a0_fd0/1(reg_in) -> Slice3/1, Slice2/1
  Slice4/1 -> Concat22/4
  Slice5/1 -> Concat22/5
  Constant27/1 -> adc_ctrl0/1(sim_reg_in)
  adc_ctrl0/1(reg_in) -> Slice29/1, Slice30/1
  Logical/1 -> edge_detect1/1
  edge_detect1/1 -> [adc_sync0]
  [mrst] -> fd_local_time/1(reset)
  fd_local_time/1(time) -> tvg_tr/4(current_time), Delay24/1
  [armed] -> Concat21/1
  Counter3/1 -> Concat21/2
  Concat21/1 -> pps_count/1(reg_out)
  Constant40/1 -> fft_shift1/1(sim_reg_in)
  fft_shift1/1(reg_in) -> Slice26/1
  Slice26/1 -> fft_wideband_real1/2(shift)
  calc_adc_sum_sq/1(sum_sq) -> Delay8/1
  decat0/1(Out1) -> calc_adc_sum_sq/1(In1)
  decat0/2(Out2) -> calc_adc_sum_sq/2(In2)
  decat0/3(Out3) -> calc_adc_sum_sq/3(In3)
  decat0/4(Out4) -> calc_adc_sum_sq/4(In4)
  [adc0] -> decat0/1(In1)
  adc_sum_sq0/1(sim_reg_out) -> Terminator/1
  [adc1] -> decat1/1(In1)
  Constant48/1 -> adc_ctrl1/1(sim_reg_in)
  adc_ctrl1/1(reg_in) -> Slice34/1, Slice33/1
  calc_adc_sum_sq1/1(sum_sq) -> Delay12/1
  decat1/1(Out1) -> calc_adc_sum_sq1/1(In1)
  decat1/2(Out2) -> calc_adc_sum_sq1/2(In2)
  decat1/3(Out3) -> calc_adc_sum_sq1/3(In3)
  decat1/4(Out4) -> calc_adc_sum_sq1/4(In4)
  adc_sum_sq1/1(sim_reg_out) -> Terminator25/1
  Constant3/1 -> board_id/1(sim_reg_in)
  board_id/1(reg_in) -> [board_id]
  arm3/1 -> posedge2/1
  posedge2/1 -> [tvg_en]
  arm4/1 -> [ct_tvg]
  arm5/1 -> [packetiser_tvg]
  arm6/1 -> [fd_fs_tvg]
  [clr_status] -> Subsystem6/1(clr)
  [xaui_ldn0] -> Subsystem6/2(xuai_ldn)
  [xaui_of0] -> Subsystem6/3(xaui_of)
  [ct_err0] -> Subsystem6/4(ct_err)
  [adc_or0] -> Subsystem6/5(adc_or)
  [fft_or0] -> Subsystem6/6(fft_or)
  [quant_or0] -> Subsystem6/7(quant_or)
  Counter8/1 -> Slice13/1
  Constant41/1 -> eq0/2(data_in)
  Constant46/1 -> eq0/3(we)
  arm7/1 -> [adc_tvg]
  pol_pack0/1(Out1) -> [adc0]
  pol_pack1/1(Out1) -> [adc1]
  coarse_delay0/1(reg_in) -> Slice38/1
  Slice2/1 -> Concat22/1
  Slice3/1 -> Concat22/2
  [mrst] -> packetiser/4(mrst_in)
  ct/4(ct_err) -> [ct_err0]
  X-engine reorder/1(sync_out) -> Recombine channels/1(sync_in)
  X-engine reorder/2(out0) -> Recombine channels/2(channel0)
  X-engine reorder/3(out1) -> Recombine channels/3(channel1)
  Recombine channels/1(sync_out) -> ct/1(sync)
  Recombine channels/2(p0) -> ct/2(p0)
  Recombine channels/3(p1) -> ct/3(p1)
  ct_tvg/1(sync_out) -> Split channels/1(sync_in)
  ct_tvg/2(p0) -> Split channels/2(p0)
  Split channels/1(sync_out) -> X-engine reorder/1(sync)
  ct_tvg/3(p1) -> Split channels/3(p1)
  Split channels/2(even) -> X-engine reorder/2(data0)
  Split channels/3(odd) -> X-engine reorder/3(data1)
  [board_id] -> packetiser/5(ant_base)
  [quant0_sync] -> ct_tvg/2(sync_in)
  [quant0_data] -> ct_tvg/3(p0_in)
  [quant1_data] -> ct_tvg/4(p1_in)
  [ct_tvg] -> Register/1
  [tvg_en] -> Register/2
  Register/1 -> ct_tvg/1(enable)
  fft_wideband_real0/2(out0) -> Delay22/1
  fft_wideband_real0/3(out1) -> Delay21/1
  fft_wideband_real1/1(sync_out) -> Delay25/1
  fft_wideband_real1/2(out0) -> Delay26/1
  fft_wideband_real1/3(out1) -> Delay27/1
  ct/1(sync_out) -> pkt_cont_tvg0/2(sync)
  ct/2(data_valid) -> pkt_cont_tvg0/3(dv)
  pkt_cont_tvg0/1(sync_out) -> packetiser/1(sync_in)
  pkt_cont_tvg0/2(dv_out) -> packetiser/2(data_valid)
  pkt_cont_tvg0/3(dout) -> packetiser/3(data_in)
  [tvg_en] -> Register1/2
  [packetiser_tvg] -> Register1/1
  Register1/1 -> pkt_cont_tvg0/1(use_tvg)
  Register2/1 -> fd_fs_tvg0/3(use_tvg), fd_fs_tvg1/1(use_tvg)
  katadc0/5(or0) -> [adc_or0]
  katadc0/1(data0_0) -> pol_pack0/1(In1)
  katadc0/2(data0_1) -> pol_pack0/2(In2)
  katadc0/3(data0_2) -> pol_pack0/3(In3)
  katadc0/4(data0_3) -> pol_pack0/4(In4)
  katadc0/6(data1_0) -> pol_pack1/1(In1)
  katadc0/7(data1_1) -> pol_pack1/2(In2)
  katadc0/8(data1_2) -> pol_pack1/3(In3)
  katadc0/9(data1_3) -> pol_pack1/4(In4)
  katadc0/10(or1) -> [adc_or1]
  katadc0/15(data_valid) -> Terminator1/1
  Pulse Generator2/1 -> katadc0/1(sim_data0)
  Pulse Generator/1 -> katadc0/2(sim_data1)
  Pulse Generator1/1 -> katadc0/3(sim_sync)
  Constant/1 -> katadc0/4(sim_data_valid)
  xaui_pack0/6(sync) -> Delay31/1(d)
  xaui_pack0/7(mrst) -> Delay34/1(d)
  xaui_pack0/5(discard) -> Delay33/1(d)
  [gbe_base_ip] -> xaui_pack0/5(base_ip)
  xaui_pack0/1(dout) -> Delay9/1(d)
  xaui_pack0/2(valid_out) -> Delay23/1(d)
  xaui_pack0/3(dest_ip) -> Delay10/1(d)
  xaui_pack0/4(eof) -> Delay11/1(d)
  Delay1/1(q) -> xaui_pack0/1(din)
  Delay3/1(q) -> xaui_pack0/2(oob)
  Delay4/1(q) -> xaui_pack0/3(valid_in)
  Delay5/1(q) -> xaui_pack0/4(linkdown_in)
  Delay31/1(q) -> [xaui_sync]
  Delay33/1(q) -> [xaui_pkt_err]
  Delay34/1(q) -> [xaui_mrst]
  [gbe_enable] -> Logical1/2
  xaui_pack0/8(hdr) -> Terminator8/1
  xaui_pack0/9(hdr_valid) -> Terminator7/1
  [xaui_data] -> Delay1/1(d)
  [xaui_oob] -> Delay3/1(d)
  [xaui_valid] -> Delay4/1(d)
  [xaui_link_dn] -> Delay5/1(d)
  Delay9/1(q) -> [xaui_pkt_data]
  Delay23/1(q) -> [xaui_pkt_valid]
  Delay10/1(q) -> [xaui_pkt_ip]
  Delay11/1(q) -> [xaui_pkt_eof]
  [xaui_pkt_valid] -> Logical1/1
  Logical2/1 -> [gbe_rst_int]
  [gbe_rst] -> Logical2/2
  Constant20/1 -> Logical2/1
  Logical1/1 -> [gbe_txvld]
  [xaui_pkt_data] -> [gbe_txd]
  [xaui_pkt_ip] -> [gbe_txip]
  [xaui_pkt_eof] -> [gbe_txeof]
  [xaui_pkt_err] -> [gbe_tx_discard]
  [gbe_rst_int] -> gbe0/1
  [gbe_txd] -> gbe0/2
  [gbe_txvld] -> gbe0/3
  [gbe_txip] -> gbe0/4
  [gbe_txport] -> gbe0/5
  [gbe_txeof] -> gbe0/6
  [gbe_rxack] -> gbe0/7
  Constant5/1 -> [gbe_rxack]
  [gbe_rxack] -> gbe0/8
  gbe0/1 -> [gbe_up], Inverter/1
  gbe0/2 -> [gbe_rx]
  gbe0/3 -> [gbe_tx]
  gbe0/4 -> [gbe_tx_full]
  gbe0/5 -> [gbe_tx_over], [xaui_of0]
  gbe0/12 -> [gbe_rx_over]
  gbe0/11 -> [gbe_rx_bad]
  gbe0/6 -> [gbe_rxdata]
  gbe0/7 -> [gbe_rxvld]
  gbe0/8 -> [gbe_rxip]
  gbe0/9 -> [gbe_rxport]
  gbe0/10 -> [gbe_rxeof]
  err_clear_sim4/1 -> gbe_port/1(sim_reg_in)
  gbe_port/1(reg_in) -> Delay71/1(d)
  err_clear_sim3/1 -> gbe_ip0/1(sim_reg_in)
  gbe_ip0/1(reg_in) -> Delay72/1(d)
  Delay71/1(q) -> [gbe_txport]
  Delay72/1(q) -> [gbe_base_ip]
  packetiser/1(data_out) -> [xaui_data]
  packetiser/2(oob) -> [xaui_oob]
  packetiser/3(valid_out) -> [xaui_valid]
  Constant7/1 -> [xaui_link_dn]
  arm8/1 -> Delay100/1(d)
  arm9/1 -> Delay2/1(d)
  Delay100/1(q) -> [gbe_enable]
  Delay2/1(q) -> [gbe_rst]
  Inverter/1 -> [xaui_ldn0]
  gbe_err/1(tx_cnt) -> Delay14/1(d)
  [clr_status] -> gbe_err/1(rst)
  gbe_err/2(err_cnt) -> Delay64/1(d)
  gbe_tx_err_cnt0/1(sim_reg_out) -> Terminator4/1
  Delay64/1(q) -> gbe_tx_err_cnt0/1(reg_out)
  [gbe_tx_discard] -> gbe_err/2(discard)
  [gbe_txeof] -> gbe_err/3(eof)
  [gbe_txvld] -> gbe_err/4(valid)
  Subsystem1/1(status) -> fstatus1/1(reg_out)
  arm10/1 -> [fancy_en]
  fft_wideband_real1/4(of) -> [fft_or1]
  [adc0] -> decat2/1(i1)
  decat2/1(o1) -> auto_shutoff0/1(In1)
  decat2/2(o2) -> auto_shutoff0/2(In2)
  decat2/3(o3) -> auto_shutoff0/3(In3)
  decat2/4(o4) -> auto_shutoff0/4(In4)
  [clr_status] -> auto_shutoff0/5(rst)
  auto_shutoff0/1(en) -> [adc_bad0]
  katadc0/11(sync0) -> Concat8/1
  katadc0/12(sync1) -> Concat8/2
  katadc0/13(sync2) -> Concat8/3
  katadc0/14(sync3) -> Concat8/4
  Concat8/1 -> [sync_raw0]
  Slice35/1 -> Logical/1
  Slice36/1 -> Logical/2
  Slice40/1 -> Logical/3
  Slice45/1 -> Logical/4
  [sync_raw0] -> Slice35/1, Slice45/1, Slice40/1, Slice36/1
  [sync_val0] -> Subsystem6/8(sync_val)
  [adc1] -> decat3/1(i1)
  decat3/1(o1) -> auto_shutoff1/1(In1)
  decat3/2(o2) -> auto_shutoff1/2(In2)
  decat3/3(o3) -> auto_shutoff1/3(In3)
  decat3/4(o4) -> auto_shutoff1/4(In4)
  [clr_status] -> auto_shutoff1/5(rst)
  auto_shutoff1/1(en) -> [adc_bad1]
  arm11/1 -> [adc_protect_disable]
  [adc_bad_latch0] -> Inverter1/1
  Inverter1/1 -> Logical6/1
  [adc_protect_disable] -> Logical6/2
  Logical8/1 -> [adc_en0], katadc0/5(en0)
  Logical6/1 -> Logical8/1
  Slice29/1 -> Logical8/2
  Slice30/1 -> katadc0/6(atten0)
  [adc_bad_latch1] -> Inverter2/1
  Inverter2/1 -> Logical9/1
  [adc_protect_disable] -> Logical9/2
  Logical9/1 -> Logical10/1
  Slice33/1 -> Logical10/2
  Logical10/1 -> [adc_en1], katadc0/7(en1)
  Slice34/1 -> katadc0/8(atten1)
  [mrst] -> edge_detect/1(in)
  edge_detect/1(out) -> Delay/1
  [mrst] -> fd_tr0/1(rst)
  Logical12/1 -> fd_tr0/3(arm)
  [fd_local_time] -> fd_tr0/4(current_time)
  Slice38/1 -> AddSub/1
  [sync_val0] -> AddSub/2
  AddSub/1 -> Concat22/3
  Constant25/1 -> trig_level/1(sim_reg_in)
  trig_level/1(reg_in) -> trig0/3(trig_pnt), trig1/3(trig_pnt)
  [data_adc1] -> trig1/2(adc_data)
  [sync_raw0] -> Register3/1
  [adc_sync0] -> Logical5/1
  Logical5/1 -> Register3/2
  [mrst] -> Logical5/2
  Register3/1 -> convert/1(in)
  convert/1(Out1) -> [sync_val0]
  gbe_tx_cnt0/1(sim_reg_out) -> Terminator3/1
  Delay14/1(q) -> gbe_tx_cnt0/1(reg_out)
  trig1/3(trig) -> [trig_adc1]
  Delay8/1 -> adc_sum_sq0/1(reg_out)
  Delay12/1 -> adc_sum_sq1/1(reg_out)
  trig1/2(data) -> [trig_data1]
  [trig_data0] -> adc_snap0/1(din)
  trig0/2(data) -> [trig_data0]
  trig0/3(trig) -> [trig_adc0]
  [sync] -> trig1/1(synci)
  [data_adc0] -> trig0/2(adc_data)
  trig1/1(synco) -> Terminator5/1
  [pps] -> Counter1/1, Counter3/2, Register19/2
  Delay7/1 -> Slice1/1, Slice/1
  Delay/1 -> [load_now]
  Slice7/1 -> [arm0]
  [arm0] -> Logical12/2
  [load_now] -> Logical12/1, fd_tr0/2(load_now)
  Concat9/1 -> [ld_time0]
  Concat22/1 -> [ld_val0]
  fd_tr0/1(arm_count) -> Convert4/1
  fd_tr0/2(ld_count) -> Convert5/1
  [ld_time0] -> fd_tr0/5(load_time)
  [ld_val0] -> fd_tr0/6(load_value)
  a1_fd0/1(reg_in) -> Slice4/1, Slice5/1
  Constant16/1 -> ld_time_msw1/1(sim_reg_in)
  Constant6/1 -> ld_time_lsw1/1(sim_reg_in)
  ld_time_msw1/1(reg_in) -> Slice51/1, Slice52/1
  Slice52/1 -> Concat12/1
  ld_time_lsw1/1(reg_in) -> Concat12/2
  Constant15/1 -> coarse_delay1/1(sim_reg_in)
  Constant31/1 -> a0_fd1/1(sim_reg_in)
  Constant26/1 -> a1_fd1/1(sim_reg_in)
  a0_fd1/1(reg_in) -> Slice46/1, Slice47/1
  Slice49/1 -> Concat11/4
  Slice50/1 -> Concat11/5
  coarse_delay1/1(reg_in) -> Slice48/1
  Slice46/1 -> Concat11/1
  Slice47/1 -> Concat11/2
  Slice48/1 -> AddSub2/1
  [sync_val0] -> AddSub2/2
  AddSub2/1 -> Concat11/3
  Slice51/1 -> [arm1]
  Concat12/1 -> [ld_time1]
  Concat11/1 -> [ld_val1]
  a1_fd1/1(reg_in) -> Slice50/1, Slice49/1
  fd_fs_tvg0/4(dout1) -> bus_create/3(in3), Subsystem4/3(din1)
  bus_create/1(bus_out) -> fd_tr0/7(din)
  fd_tr0/5(dout) -> bus_expand1/1(bus_in)
  bus_expand1/2(out2) -> Delay15/1
  bus_expand1/3(lsb_out1) -> Delay16/1
  bus_expand1/1(msb_out3) -> Counter8/1, Delay13/1
  eq0/1(data_out) -> bus_expand2/1(bus_in)
  Delay13/1 -> quant0/5(sync_in)
  Delay15/1 -> quant0/6(din0)
  Delay16/1 -> quant0/7(din1)
  bus_expand2/1(msb_out4) -> quant0/1(real_in0)
  bus_expand2/2(out3) -> quant0/2(imag_in0)
  bus_expand2/3(out2) -> quant0/3(real_in1)
  bus_expand2/4(lsb_out1) -> quant0/4(real_in2)
  [mrst] -> fd_tr1/1(rst)
  Logical13/1 -> fd_tr1/3(arm)
  [fd_local_time] -> fd_tr1/4(current_time)
  [arm1] -> Logical13/2
  [load_now] -> fd_tr1/2(load_now), Logical13/1
  fd_tr1/1(arm_count) -> Convert6/1
  fd_tr1/2(ld_count) -> Convert7/1
  [ld_time1] -> fd_tr1/5(load_time)
  [ld_val1] -> fd_tr1/6(load_value)
  fd_fs_tvg1/1(sync_out) -> bus_create1/1(in1)
  fd_fs_tvg1/2(dout0) -> bus_create1/2(in2)
  fd_fs_tvg1/3(dout1) -> bus_create1/3(in3)
  bus_create1/1(bus_out) -> fd_tr1/7(din)
  fd_tr1/5(dout) -> bus_expand3/1(bus_in)
  quant1/2(dout0) -> Concat3/1
  quant1/3(dout1) -> Concat3/2
  quant1/1(sync_out) -> [quant1_sync]
  quant1/4(of) -> [quant_or1]
  Concat3/1 -> [quant1_data]
  bus_expand3/1(msb_out3) -> Delay17/1, Counter2/1
  Counter2/1 -> Slice25/1
  Constant10/1 -> eq1/2(data_in)
  Constant11/1 -> eq1/3(we)
  bus_expand3/2(out2) -> Delay18/1
  bus_expand3/3(lsb_out1) -> Delay19/1
  eq1/1(data_out) -> bus_expand4/1(bus_in)
  Delay17/1 -> quant1/5(sync_in)
  Delay18/1 -> quant1/6(din0)
  Delay19/1 -> quant1/7(din1)
  bus_expand4/1(msb_out4) -> quant1/1(real_in0)
  bus_expand4/2(out3) -> quant1/2(imag_in0)
  bus_expand4/3(out2) -> quant1/3(real_in1)
  bus_expand4/4(lsb_out1) -> quant1/4(real_in2)
  [mrst] -> gpio_tr/1(rst)
  Logical15/1 -> gpio_tr/3(arm)
  [cd_local_time] -> gpio_tr/4(current_time)
  [load_now] -> gpio_tr/2(load_now), Logical15/1
  [arm0] -> Logical15/2
  [ld_time0] -> gpio_tr/5(load_time)
  Constant34/1 -> gpio_tr/6(load_value)
  [timing_pps] -> gpio_tr/7(din)
  gpio_tr/5(dout) -> Delay28/1
  gpio_tr/1(arm_count) -> Terminator19/1
  gpio_tr/2(ld_count) -> Terminator21/1
  Constant24/1 -> adc_snap0/2(we)
  [trig_sync] -> adc_snap0/3(trig)
  [trig_adc0] -> adc_snap0/4(stop)
  [timing_pps] -> Concat6/1
  [cd_local_time] -> Slice14/1
  Concat6/1 -> trig0/1(synci)
  trig0/1(synco) -> bus_expand/1(bus_in)
  bus_expand/1(msb_out2) -> [trig_sync]
  Slice14/1 -> Concat6/2
  bus_expand/2(lsb_out1) -> [timestamp]
  [timestamp] -> adc_snap0/5(vin)
  [trig_data1] -> adc_snap1/1(din)
  [trig_adc1] -> adc_snap1/4(stop)
  [timestamp] -> adc_snap1/5(vin)
  [trig_sync] -> scopes/1(sync)
  [timestamp] -> scopes/3(time)
  [trig_data0] -> scopes/2(data)
  [adc_en0] -> Inverter5/1
  [adc_en1] -> Inverter6/1
  fd_fs_tvg0/3(dout0) -> bus_create/2(in2), Subsystem4/2(din0)
  fd_fs_tvg0/2(sync_out) -> bus_create/1(in1), Subsystem4/1(sync)
  fd_fs_tvg0/1(time_o) -> [fd_local_time]
  [fd_local_time] -> Subsystem4/4(time)
  [mrst] -> tvg_tr/1(rst)
  Logical20/1 -> tvg_tr/3(arm)
  [arm0] -> Logical20/2
  [load_now] -> tvg_tr/2(load_now), Logical20/1
  [ld_time0] -> tvg_tr/5(load_time)
  Constant35/1 -> tvg_tr/6(load_value)
  Constant14/1 -> tvg_tr/7(din)
  tvg_tr/5(dout) -> Terminator23/1
  tvg_tr/1(arm_count) -> Terminator26/1
  tvg_tr/2(ld_count) -> Terminator27/1
  tvg_tr/3(value) -> Terminator29/1
  tvg_tr/4(enable) -> [tvg_tr_en]
  [fd_fs_tvg] -> Register2/1
  Delay20/1 -> fd_fs_tvg0/2(sync)
  Delay21/1 -> fd_fs_tvg0/5(din1)
  Delay22/1 -> fd_fs_tvg0/4(din0)
  Delay24/1 -> fd_fs_tvg0/1(time)
  Delay25/1 -> fd_fs_tvg1/2(sync)
  Delay26/1 -> fd_fs_tvg1/3(din0)
  Delay27/1 -> fd_fs_tvg1/4(din1)
  Logical7/1 -> leds/1(warning)
  [sync] -> leds/4(sync_led)
  Logical4/1 -> leds/6(comms)
  [pps] -> leds/5(pps)
  [mrst] -> leds/3(rst)
  [fancy_en] -> leds/2(fancy_en)
  [xaui_ldn0] -> Logical4/1
  [xaui_of0] -> Logical4/2
  [adc_en0] -> Inverter3/1
  [adc_en1] -> Inverter4/1
  [adc_or1] -> Logical16/1
  [fft_or1] -> Logical16/2
  [adc_or0] -> Logical17/1
  [fft_or0] -> Logical17/2
  Logical17/1 -> leds/9(or0)
  Logical16/1 -> leds/10(or1)
  [adc_en0] -> leds/7(adc_en0)
  [adc_en1] -> leds/8(adc_en1)
  [xaui_of0] -> Logical7/2
  [xaui_ldn0] -> Logical7/1
  [adc_sync0] -> adc_scope/1(In1)
  [adc0] -> adc_scope/2(In2)
  [adc1] -> adc_scope/3(In3)
  gpio_tr/3(value) -> Terminator28/1
  gpio_tr/4(enable) -> Register4/2
  [tvg_en] -> Register2/2
  [tvg_tr_en] -> fd_fs_tvg0/6(val_en)
  Constant36/1 -> Relational/2
  Constant37/1 -> Relational1/2
  Relational/1 -> Logical21/1
  Relational1/1 -> Logical21/2
  Logical21/1 -> [clk_bad]
  [clk_bad] -> Subsystem6/10(clk_bad)
  Inverter5/1 -> Subsystem6/9(adc_disable)
  [clr_status] -> Subsystem1/1(clr)
  [xaui_ldn0] -> Subsystem1/2(xuai_ldn)
  [xaui_of0] -> Subsystem1/3(xaui_of)
  [ct_err0] -> Subsystem1/4(ct_err)
  [adc_or1] -> Subsystem1/5(adc_or)
  [fft_or1] -> Subsystem1/6(fft_or)
  [quant_or1] -> Subsystem1/7(quant_or)
  [sync_val0] -> Subsystem1/8(sync_val)
  Inverter6/1 -> Subsystem1/9(adc_disable)
  [clk_bad] -> Subsystem1/10(clk_bad)
  [trig_sync] -> adc_snap1/3(trig)
  Constant1/1 -> adc_snap1/2(we)
  [arm0] -> edge_detect2/1(in)
  Constant38/1 -> Register4/1
  Register4/1 -> Subsystem5/1(In1)
  edge_detect2/1(out) -> Register4/3
  [adc0] -> Concat/2
  [adc1] -> Concat/3
  [adc_sync0] -> Concat/1
  timing/3(data_out) -> bus_expand6/1(bus_in)
  bus_expand6/3(lsb_out1) -> [data_adc1]
  bus_expand6/2(out2) -> [data_adc0]
  bus_expand6/1(msb_out3) -> [timing_pps]
  Delay28/1 -> Subsystem5/2(din)
  Inverter3/1 -> Logical7/3
  Inverter4/1 -> Logical7/4
  [clk_bad] -> Logical7/5
  delay_tr_status0/1(sim_reg_out) -> Terminator17/1
  Convert4/1 -> Concat13/1
  Concat13/1 -> delay_tr_status0/1(reg_out)
  Convert5/1 -> Concat13/2
  delay_tr_status1/1(sim_reg_out) -> Terminator6/1
  Convert6/1 -> Concat14/1
  Concat14/1 -> delay_tr_status1/1(reg_out)
  Convert7/1 -> Concat14/2
  Constant13/1 -> Subsystem1/11(dram_bad)
  Constant12/1 -> Subsystem6/11(dram_bad)
  Subsystem6/1(status) -> fstatus0/1(reg_out)
  Register9/1 -> [adc_bad_latch0]
  [ct_err0] -> Logical7/7
  Constant17/1 -> Logical7/6
  Slice13/1 -> eq0/1(addr)
  Slice25/1 -> eq1/1(addr)
  [sync] -> pfb_fir_real0/1(sync)
  [data_adc0] -> bus_expand7/1(bus_in)
  [data_adc1] -> bus_expand5/1(bus_in)
  [gbe_up] -> Subsystem3/5(link_up)
  [gbe_tx] -> Subsystem3/6(led_tx)
  [gbe_txd] -> Subsystem3/1(data_in)
  [gbe_txvld] -> Subsystem3/2(valid_in)
  [gbe_txip] -> Subsystem3/3(ip_in)
  [gbe_txeof] -> Subsystem3/4(eof_in)
  [gbe_tx_full] -> Subsystem3/7(tx_full)
  [gbe_tx_over] -> Subsystem3/8(tx_over)
  Subsystem3/1(data_out) -> snap_gbe_tx0/1(din)
  Subsystem3/2(we_out) -> snap_gbe_tx0/2(we)
  [board_id] -> pkt_cont_tvg0/5(antenna)
  ct/3(data_out) -> pkt_cont_tvg0/4(din)
  [adc_bad0] -> Register9/1, Register9/3
  [clr_status] -> Register9/2
  Register5/1 -> [adc_bad_latch1]
  [adc_bad1] -> Register5/3, Register5/1
  [clr_status] -> Register5/2
  [xaui_sync] -> snap_gbe_tx0/3(trig)
